simulink model "CCMVgaMotor"  (R2022b)
settings: Solver=FixedStepDiscrete  StartTime=0.0  StopTime=10.0  FixedStep=0.01  RelTol=1e-3
model workspace (MAT data in the model file):
  NvMBlockVgaMtrCalData = Simulink.Signal
  NvMBlockVgaMtrCalData.DataType = 'UInt8'
  NvMBlockVgaMtrCalData.Dimensions = [210 1]
  NvMBlockVgaMtrCalData.DimensionsMode = 'auto'
  NvMBlockVgaMtrCalData.Complexity = 'real'
  NvMBlockVgaMtrCalData.SampleTime = -1
  NvMBlockVgaMtrCalData.SamplingMode = 'auto'
  NvMBlockVgaMtrCalData.InitialValue = 'NvMBlockVgaMtrCalDataInit'
  NvMBlockVgaMtrCalDataInit = Simulink.Parameter
  NvMBlockVgaMtrCalDataInit.Value = [0; 0; 0; 0; 0; 0; 0; 0; 0; 0; 0; 0; 0; 0; 0; 0; 0; 0; 0; 0; 0; 0; 0; 0; 0; 0; 0; 0; 0; 0; 0; 0; 0; 0; 0; 0; 0; 0; 0; 0; 0; 0; 0; 0; 0; 0; 0; 0; 0; 0; 0; 0; 0; 0; 0; 0; 0; 0; 0; 0; 0; 0; 0; 0; 0; 0; 0; 0; 0; 0; 0; 0; 0; 0; 0; 0; 0; 0; 0; 0; 0; 0; 0; 0; 0; 0; 0; 0; 0; 0; 0; 0; 0; 0; 0; 0; 0; 0; 0; 0; 0; 0; 0; 0; 0; 0; 0; 0; 0; 0; 0; 0; 0; 0; 0; 0; 0; 0; 0; 0; 0; 0; 0; 0; 0; 0; 0; 0; 0; 0; 0; 0; 0; 0; 0; 0; 0; 0; 0; 0; 0; 0; 0; 0; 0; 0; 0; 0; 0; 0; 0; 0; 0; 0; 0; 0; 0; 0; 0; 0; 0; 0; 0; 0; 0; 0; 0; 0; 0; 0; 0; 0; 0; 0; 0; 0; 0; 0; 0; 0; 0; 0; 0; 0; 0; 0; 0; 0; 0; 0; 0; 0; 0; 0; 0; 0; 0; 0; 0; 0; 0; 0; 0; 0; 0; 0; 0; 0; 0; 0]
  NvMBlockVgaMtrCalDataInit.DataType = 'UInt8'
  NvMBlockVgaMtrCalDataInit.Complexity = 'real'
  NvMBlockVgaMtrCalDataInit.Dimensions = [210 1]
  VgaWakeUpFlag = Simulink.Signal
  VgaWakeUpFlag.DataType = 'UInt8'
  VgaWakeUpFlag.Dimensions = 1
  VgaWakeUpFlag.DimensionsMode = 'auto'
  VgaWakeUpFlag.Complexity = 'real'
  VgaWakeUpFlag.SampleTime = -1
  VgaWakeUpFlag.SamplingMode = 'auto'
undefined symbols (not defined in the file or in its folder): ComChlType, DioChannelType, LinFrameType, NetworkChlType, NvMBlockId, P_DioLevelType, P_LinIfSchHandleType, P_Std_ReturnType, PowerChlType, VGAChlType
(part 1 of 4)
settings Model: Creator=blue.ge  LastModifiedBy=blue.ge
settings DataIOCC: SaveFormat=StructureWithTime
settings OptimizationCC: DefaultParameterBehavior=Inlined  EnhancedBackFolding=on  PassReuseOutputArgsAs=Individual arguments  ZeroExternalMemoryAtStartup=off  ZeroInternalMemoryAtStartup=off  OptimizeBlockOrder=speed  DifferentSizesBufferReuse=on  OptimizationCustomize=off  EfficientTunableParamExpr=on
settings DebuggingCC: ParameterTunabilityLossMsg=error
settings ModelReferenceCC: ModelReferenceNumInstancesAllowed=Single
settings SFSimCC: MATLABDynamicMemAlloc=off
settings RTWCC: Description=AUTOSAR  SystemTargetFile=autosar.tlc  GenCodeOnly=on  TemplateMakefile=ert_default_tmf
settings CodeAppCC: ForceParamTrailComments=on  IgnoreCustomStorageClasses=off  OperatorAnnotations=on  SimulinkDataObjDesc=on  SFDataObjDesc=on  InsertBlockDesc=on
settings STFCustomTargetCC: TargetLangStandard=C99 (ISO)  MultiwordLength=256  ParMdlRefBuildCompliant=on  CompOptLevelCompliant=on  IncludeMdlTerminateFcn=off  CombineOutputUpdateFcns=on  SuppressErrorStatus=on  ERTCustomFileBanners=on  SupportNonFinite=off  SupportComplex=off  SupportContinuousTime=off  SupportNonInlinedSFcns=off  RemoveResetFunc=on  MATLABClassNameForMDSCustomization=Simulink.SoftwareTarget.ERTCustomization  AutosarCompliant=on  UseToolchainInfoCompliant=on  LookupTableObjectStructAxisOrder=2,1,3,4,...  LUTObjectStructOrderEvenSpacing=Size,Table,Breakpoints  SystemTargetFile=autosar.tlc
settings ERTTargetCC: TargetLangStandard=C99 (ISO)  ConcurrentExecutionCompliant=off  IncludeMdlTerminateFcn=off  SuppressErrorStatus=on  SupportNonFinite=off  SupportComplex=off  ModelStepFunctionPrototypeControlCompliant=off  CPPClassGenCompliant=off  AutosarCompliant=on  LookupTableObjectStructAxisOrder=2,1,3,4,...  LUTObjectStructOrderEvenSpacing=Size,Table,Breakpoints  InlinedParameterPlacement=NonHierarchical  ERTCustomFileTemplate=example_file_process.tlc

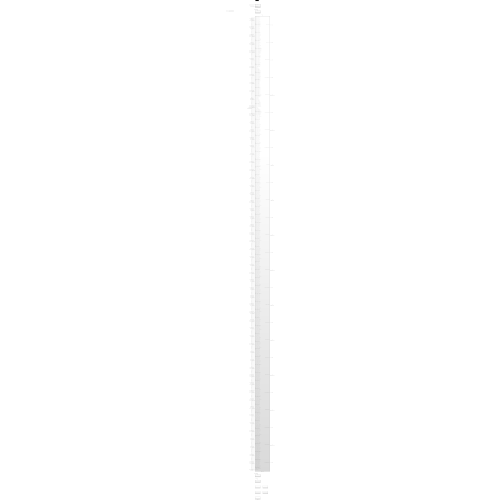
[thumbnail: Simulink's stored preview of the top-level diagram]
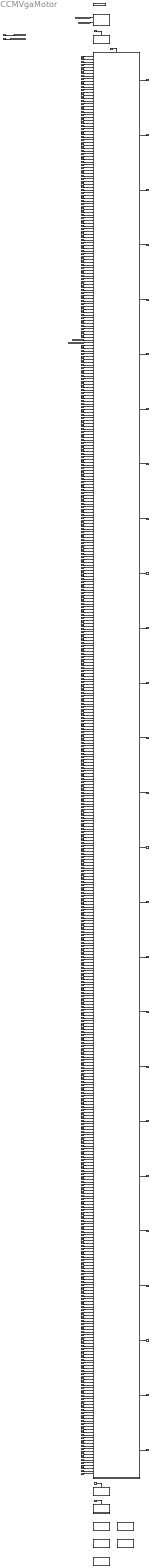
== system CCMVgaMotor ==
  CCMVgaMotor_50msRunnable : Inport  SampleTime=0.05  OutputFunctionCall=on
  CCMVgaMotor_Runnable : Inport  Port=2  SampleTime=0.02  OutputFunctionCall=on
  CCMVGAMotor_VentnMotAdr : Inport  Port=3  OutputFunctionCall=on
  CCMVGAMotor_VentnMotAdr2 : Inport  Port=4  OutputFunctionCall=on
  SbcWakeupSta_SbcWakeupSta : Inport  Port=5  OutDataTypeStr=Enum: SbcWakeupSta  PortDimensions=1
  SbcPwrOn_SbcPwrOn : Inport  Port=6  OutDataTypeStr=PwrOnff  PortDimensions=1
  CarCfgForClimaCtrl_CarCfgForAirClng : Inport  Port=7  OutDataTypeStr=Enum: CarCfgForAirClng  PortDimensions=1
  CarCfgForClimaCtrl_CarCfgForAirClnrAdv : Inport  Port=8  OutDataTypeStr=UInt8  PortDimensions=1
  CarCfgForClimaCtrl_CarCfgForAirFrag : Inport  Port=9  OutDataTypeStr=Enum: CarCfgForAirFrag  PortDimensions=1
  CarCfgForClimaCtrl_CarCfgForAirMtrlSnsr : Inport  Port=10  OutDataTypeStr=Enum: CarCfgForAirMtrlSnsrGly  PortDimensions=1
  CarCfgForClimaCtrl_CarCfgForAirQly : Inport  Port=11  OutDataTypeStr=Enum: CarCfgForAirQlyGly  PortDimensions=1
  CarCfgForClimaCtrl_CarCfgForAmntOfSeat : Inport  Port=12  OutDataTypeStr=Enum: CarCfgForAmntOfSeatTyp3  PortDimensions=1
  CarCfgForClimaCtrl_CarCfgForAuxWtrPmp : Inport  Port=13  OutDataTypeStr=Enum: CarCfgForAuxWtrPmp  PortDimensions=1
  CarCfgForClimaCtrl_CarCfgForBypVlvInHvac : Inport  Port=14  OutDataTypeStr=Enum: CarCfgForBypVlvInHvac2  PortDimensions=1
  CarCfgForClimaCtrl_CarCfgForClsVlvInHvac : Inport  Port=15  OutDataTypeStr=Enum: CarCfgForClsVlvInHvac  PortDimensions=1
  CarCfgForClimaCtrl_CarCfgForCmprAcTyp : Inport  Port=16  OutDataTypeStr=UInt8  PortDimensions=1
  CarCfgForClimaCtrl_CarCfgForCmptmtClng : Inport  Port=17  OutDataTypeStr=Enum: CarCfgForCmptmtClng  PortDimensions=1
  CarCfgForClimaCtrl_CarCfgForElecVentAdjmt : Inport  Port=18  OutDataTypeStr=Enum: CarCfgForElecVentAdjmt  PortDimensions=1
  CarCfgForClimaCtrl_CarCfgForFlcTyp : Inport  Port=19  OutDataTypeStr=Enum: CarCfgForFlcTyp  PortDimensions=1
  CarCfgForClimaCtrl_CarCfgForFoldCSD : Inport  Port=20  OutDataTypeStr=Enum: CarCfgForFoldCSD  PortDimensions=1
  CarCfgForClimaCtrl_CarCfgForFuTyp : Inport  Port=21  OutDataTypeStr=Enum: CarCfgForFuTyp  PortDimensions=1
  CarCfgForClimaCtrl_CarCfgForGlassTyp : Inport  Port=22  OutDataTypeStr=Enum: CarCfgForGlassTyp  PortDimensions=1
  CarCfgForClimaCtrl_CarCfgForHeatPmp : Inport  Port=23  OutDataTypeStr=Enum: CarCfgForHeatPmpTyp  PortDimensions=1
  CarCfgForClimaCtrl_CarCfgForHeatrDurgDrvg : Inport  Port=24  OutDataTypeStr=Enum: CarCfgForHeatrDurgDrvg  PortDimensions=1
  CarCfgForClimaCtrl_CarCfgForHeatrTyp : Inport  Port=25  OutDataTypeStr=UInt8  PortDimensions=1
  CarCfgForClimaCtrl_CarCfgForHvacFilTyp : Inport  Port=26  OutDataTypeStr=Enum: CarCfgForHvacFilTypGly  PortDimensions=1
  CarCfgForClimaCtrl_CarCfgForHvacFlrSnsrForT : Inport  Port=27  OutDataTypeStr=Enum: CarCfgForHvacFlrSnsrForT  PortDimensions=1
  CarCfgForClimaCtrl_CarCfgForHvacHeatrSnsrT : Inport  Port=28  OutDataTypeStr=Enum: CarCfgForHvacHeatrSnsrT  PortDimensions=1
  CarCfgForClimaCtrl_CarCfgForHvacRe : Inport  Port=29  OutDataTypeStr=UInt8  PortDimensions=1
  CarCfgForClimaCtrl_CarCfgForHvacTyp : Inport  Port=30  OutDataTypeStr=UInt8  PortDimensions=1
  CarCfgForClimaCtrl_CarCfgForLoVoltElecCfg : Inport  Port=31  OutDataTypeStr=Enum: CarCfgForLoVoltElecCfg  PortDimensions=1
  CarCfgForClimaCtrl_CarCfgForNavSysandIntnmap : Inport  Port=32  OutDataTypeStr=Enum: CarCfgForNavSysandIntnmap  PortDimensions=1
  CarCfgForClimaCtrl_CarCfgForNrOfDoor : Inport  Port=33  OutDataTypeStr=Enum: CarCfgForNrOfDoor2  PortDimensions=1
  CarCfgForClimaCtrl_CarCfgForPipeInFuTank : Inport  Port=34  OutDataTypeStr=Enum: CarCfgForPipeInFuTank  PortDimensions=1
  CarCfgForClimaCtrl_CarCfgForPreClng : Inport  Port=35  OutDataTypeStr=Enum: CarCfgForPreClng  PortDimensions=1
  CarCfgForClimaCtrl_CarCfgForPrkgCDSNotAvl : Inport  Port=36  OutDataTypeStr=Enum: CarCfgForPrkgCDSNotAvl  PortDimensions=1
  CarCfgForClimaCtrl_CarCfgForPrkgClima : Inport  Port=37  OutDataTypeStr=Enum: CarCfgForPrkgClima  PortDimensions=1
  CarCfgForClimaCtrl_CarCfgForPrpsnTyp : Inport  Port=38  OutDataTypeStr=Enum: CarCfgForPrpsnTypGly  PortDimensions=1
  CarCfgForClimaCtrl_CarCfgForRemEngStrtPreCdn : Inport  Port=39  OutDataTypeStr=Enum: CarCfgForRemEngStrtPreCdn  PortDimensions=1
  CarCfgForClimaCtrl_CarCfgForRoofHatch : Inport  Port=40  OutDataTypeStr=Enum: CarCfgForRoofHatch  PortDimensions=1
  CarCfgForClimaCtrl_CarCfgForSeatHeatgCal : Inport  Port=41  OutDataTypeStr=Enum: CarCfgForSeatHeatgCal1  PortDimensions=1
  CarCfgForClimaCtrl_CarCfgForSeatHeatgForRowFirst : Inport  Port=42  OutDataTypeStr=Enum: CarCfgForSeatHeatgForRowFirst  PortDimensions=1
  CarCfgForClimaCtrl_CarCfgForSeatHeatgForRowSec : Inport  Port=43  OutDataTypeStr=Enum: CarCfgForSeatHeatgForRowSec  PortDimensions=1
  CarCfgForClimaCtrl_CarCfgForSeatVentnForRowFirst : Inport  Port=44  OutDataTypeStr=Enum: CarCfgForSeatVentnForRowFirst1  PortDimensions=1
  CarCfgForClimaCtrl_CarCfgForSeatVentnForRowSec : Inport  Port=45  OutDataTypeStr=Enum: CarCfgForSeatVentnForRowSecGly  PortDimensions=1
  CarCfgForClimaCtrl_CarCfgForSecRowVentnCenter : Inport  Port=46  OutDataTypeStr=Enum: CarCfgForSecRowVentnCenter  PortDimensions=1
  CarCfgForClimaCtrl_CarCfgForSecRowVentnSide : Inport  Port=47  OutDataTypeStr=Enum: CarCfgForSecRowVentnSide  PortDimensions=1
  CarCfgForClimaCtrl_CarCfgForSmallFlowCyc : Inport  Port=48  OutDataTypeStr=Enum: CarCfgForSmallFlowCyc  PortDimensions=1
  CarCfgForClimaCtrl_CarCfgForSmkSnsr : Inport  Port=49  OutDataTypeStr=Enum: CarCfgForSmkSnsr  PortDimensions=1
  CarCfgForClimaCtrl_CarCfgForSnsrForCmptmtT : Inport  Port=50  OutDataTypeStr=Enum: CarCfgForSnsrForCmptmtT  PortDimensions=1
  CarCfgForClimaCtrl_CarCfgForSnsrHumPrsnt : Inport  Port=51  OutDataTypeStr=Enum: CarCfgForSnsrHumPrsnt  PortDimensions=1
  CarCfgForClimaCtrl_CarCfgForSteerWhlHeatg : Inport  Port=52  OutDataTypeStr=UInt8  PortDimensions=1
  CarCfgForClimaCtrl_CarCfgForSteerWhlPosn : Inport  Port=53  OutDataTypeStr=Enum: CarCfgForSteerWhlPosn  PortDimensions=1
  CarCfgForClimaCtrl_CarCfgForSunSnsr : Inport  Port=54  OutDataTypeStr=Enum: CarCfgForSunSnsr  PortDimensions=1
  CarCfgForClimaCtrl_CarCfgForTelmNodTyp : Inport  Port=55  OutDataTypeStr=Enum: CarCfgForTelmNodTyp  PortDimensions=1
  CarCfgForClimaCtrl_CarCfgForThermMngt : Inport  Port=56  OutDataTypeStr=Enum: CarCfgForThermMngt  PortDimensions=1
  CarCfgForClimaCtrl_CarCfgForVehTyp : Inport  Port=57  OutDataTypeStr=Enum: CarCfgForVehTyp3  PortDimensions=1
  CarCfgForClimaCtrl_CarCfgForVgaMotorExistSts : Inport  Port=58  OutDataTypeStr=CarCfgForVgaMotorExistSts  PortDimensions=1
  CarCfgForClimaCtrl_CarCfgForWindHeatd : Inport  Port=59  OutDataTypeStr=Enum: CarCfgForWindHeatd  PortDimensions=1
  CarCfgForClimaCtrl_CarCfgForAutDemst : Inport  Port=60  OutDataTypeStr=UInt8  PortDimensions=1
  CarCfgForClimaCtrl_CarCfgForExtVentnClimaCtrl : Inport  Port=61  OutDataTypeStr=Enum: CarCfgForExtVentnClimaCtrl  PortDimensions=1
  CarCfgForClimaCtrl_CarCfgForStartDevice : Inport  Port=62  OutDataTypeStr=Enum: StartDevice  PortDimensions=1
  HmiElecAirSwngReq_HmiElecAirSwngReq : Inport  Port=63  OutDataTypeStr=Bus: HmiElecAirSwngReq  PortDimensions=1  BusOutputAsStruct=on
  LIN2ScheTableReq_Val : Inport  Port=64  OutDataTypeStr=UInt8  PortDimensions=1
  SysServCarCfgSts_SysServCarID : Inport  Port=65  OutDataTypeStr=Enum: SysServCarID  PortDimensions=1
  SysServCarCfgSts_SysServCCPAQSSts : Inport  Port=66  OutDataTypeStr=UInt8  PortDimensions=1
  SysServCarCfgSts_SysServCCPHEXSensorSts : Inport  Port=67  OutDataTypeStr=UInt8  PortDimensions=1
  SysServCarCfgSts_SysServCCPLin2AFUSts : Inport  Port=68  OutDataTypeStr=Boolean  PortDimensions=1
  SysServCarCfgSts_SysServCCPLin2AIUSts : Inport  Port=69  OutDataTypeStr=UInt8  PortDimensions=1
  SysServCarCfgSts_SysServCCPLin2CDSSts : Inport  Port=70  OutDataTypeStr=Boolean  PortDimensions=1
  SysServCarCfgSts_SysServCCPLin2PMSISts : Inport  Port=71  OutDataTypeStr=Boolean  PortDimensions=1
  SysServCarCfgSts_SysServCCPLin3VGASts : Inport  Port=72  OutDataTypeStr=Boolean  PortDimensions=1
  SysServCarCfgSts_SysServDUAirVentSnrSts : Inport  Port=73  OutDataTypeStr=Boolean  PortDimensions=1
  SysServCarCfgSts_SysServDUBlowerPwmSignSts : Inport  Port=74  OutDataTypeStr=Boolean  PortDimensions=1
  SysServCarCfgSts_SysServDUBlowerPwmSts : Inport  Port=75  OutDataTypeStr=Boolean  PortDimensions=1
  SysServCarCfgSts_SysServDUDCDefrostSts : Inport  Port=76  OutDataTypeStr=UInt8  PortDimensions=1
  SysServCarCfgSts_SysServDUDCModeSts : Inport  Port=77  OutDataTypeStr=UInt8  PortDimensions=1
  SysServCarCfgSts_SysServDUDCRecSts : Inport  Port=78  OutDataTypeStr=UInt8  PortDimensions=1
  SysServCarCfgSts_SysServDUDCSModeSts : Inport  Port=79  OutDataTypeStr=UInt8  PortDimensions=1
  SysServCarCfgSts_SysServDUDCSTLSts : Inport  Port=80  OutDataTypeStr=UInt8  PortDimensions=1
  SysServCarCfgSts_SysServDUDCTLSts : Inport  Port=81  OutDataTypeStr=UInt8  PortDimensions=1
  SysServCarCfgSts_SysServDUDCTRSts : Inport  Port=82  OutDataTypeStr=UInt8  PortDimensions=1
  SysServCarCfgSts_SysServDUFLFootSts : Inport  Port=83  OutDataTypeStr=UInt8  PortDimensions=1
  SysServCarCfgSts_SysServDUFLVentSts : Inport  Port=84  OutDataTypeStr=UInt8  PortDimensions=1
  SysServCarCfgSts_SysServDUFRFootSts : Inport  Port=85  OutDataTypeStr=UInt8  PortDimensions=1
  SysServCarCfgSts_SysServDUFRVentSts : Inport  Port=86  OutDataTypeStr=UInt8  PortDimensions=1
  SysServCarCfgSts_SysServDUIncarMotorSts : Inport  Port=87  OutDataTypeStr=Boolean  PortDimensions=1
  SysServCarCfgSts_SysServDULin1HBMFSts : Inport  Port=88  OutDataTypeStr=Boolean  PortDimensions=1
  SysServCarCfgSts_SysServDULin4HFAFSts : Inport  Port=89  OutDataTypeStr=UInt32  PortDimensions=1
  SysServCarCfgSts_SysServDUSFLFootSts : Inport  Port=90  OutDataTypeStr=UInt8  PortDimensions=1
  SysServCarCfgSts_SysServDUSFLVentSts : Inport  Port=91  OutDataTypeStr=UInt8  PortDimensions=1
  SysServCarCfgSts_SysServDUSFRFootSts : Inport  Port=92  OutDataTypeStr=UInt8  PortDimensions=1
  SysServCarCfgSts_SysServDUSFRVentSts : Inport  Port=93  OutDataTypeStr=UInt8  PortDimensions=1
  SysServCarCfgSts_SysServDUSnsrForFCmptmtTSts : Inport  Port=94  OutDataTypeStr=UInt8  PortDimensions=1
  SysServCarCfgSts_SysServDUSnsrForRair : Inport  Port=95  OutDataTypeStr=UInt8  PortDimensions=1
  SysServCarCfgSts_SysServDUSnsrForRCmptmtTSts : Inport  Port=96  OutDataTypeStr=UInt8  PortDimensions=1
  VehModMngtGlbSafe1_VehModMngtGlbSafe1 : Inport  Port=97  OutDataTypeStr=Bus: VehModMngtGlbSafe1  PortDimensions=1  BusOutputAsStruct=on
  BatLoadBtrMSts_BatLoadBtrMSts : Inport  Port=98  OutDataTypeStr=Enum: BatLoadBtrMStsEnum  PortDimensions=1
  BatSysBtrMSts_BatSysBtrMSts : Inport  Port=99  OutDataTypeStr=Enum: BatBtrMStsEnum  PortDimensions=1
  VgaMotSetPowerOnInit_PowerOnInit : Inport  Port=100  OutDataTypeStr=Boolean  PortDimensions=1
  CarCfgForClimaCtrl_CarCfgForBRAND : Inport  Port=101  OutDataTypeStr=UInt8  PortDimensions=1
  CarCfgForClimaCtrl_CarCfgForHeadRadar : Inport  Port=102  OutDataTypeStr=Enum: HeadRadar  PortDimensions=1
  CarCfgForClimaCtrl_CarCfgForInteriorViewCamera : Inport  Port=103  OutDataTypeStr=Enum: CarCfgForInteriorViewCamera  PortDimensions=1
  CarCfgForClimaCtrl_CarCfgForLifeDetection : Inport  Port=104  OutDataTypeStr=Enum: LifeDetection  PortDimensions=1
  CarCfgForClimaCtrl_CarCfgForPowerSeats : Inport  Port=105  OutDataTypeStr=Enum: CarCfgForPowerSeats  PortDimensions=1
  CarCfgForClimaCtrl_CarCfgForPTCInFrontHVAC : Inport  Port=106  OutDataTypeStr=UInt8  PortDimensions=1
  CarCfgForClimaCtrl_CarCfgForVentLiftMotorExiSts : Inport  Port=107  OutDataTypeStr=UInt32  PortDimensions=1
  CarCfgForClimaCtrl_CarCfgForWadingAssistSystem : Inport  Port=108  OutDataTypeStr=UInt8  PortDimensions=1
  SysServCarCfgSts_SysServCCPBGMNum : Inport  Port=109  OutDataTypeStr=UInt8  PortDimensions=1
  SysServCarCfgSts_SysServCCPLin2AQSSts : Inport  Port=110  OutDataTypeStr=Boolean  PortDimensions=1
  SysServCarCfgSts_SysServDUBlowerPwmSignTypeSts : Inport  Port=111  OutDataTypeStr=UInt8  PortDimensions=1
  SysServCarCfgSts_SysServDUDCFLMdeSts : Inport  Port=112  OutDataTypeStr=UInt8  PortDimensions=1
  SysServCarCfgSts_SysServDUReBlowerPwmSignSts : Inport  Port=113  OutDataTypeStr=UInt8  PortDimensions=1
  SysServCarCfgSts_SysServDUReBlowerPwmSts : Inport  Port=114  OutDataTypeStr=UInt8  PortDimensions=1
  SysServCarCfgSts_SysServLowSideRETXVSts : Inport  Port=115  OutDataTypeStr=UInt8  PortDimensions=1
  VentnActr01ActPson_VentnActr01ActPson : Inport  Port=116  OutDataTypeStr=UInt16  PortDimensions=1
  VentnActr01ActPson_VentnActr01ActPson_IsUpdated : Inport  Port=117  OutDataTypeStr=boolean  PortDimensions=1
  VentnActr01BlckInd_VentnActr01BlckInd : Inport  Port=118  OutDataTypeStr=Enum: VentnActr01BlckInd  PortDimensions=1
  VentnActr01BlckSts_VentnActr01BlckSts : Inport  Port=119  OutDataTypeStr=Enum: VentnActr01BlckSts  PortDimensions=1
  VentnActr01BusAdr_VentnActr01BusAdr : Inport  Port=120  OutDataTypeStr=UInt8  PortDimensions=1
  VentnActr01CoilSts_VentnActr01CoilSts : Inport  Port=121  OutDataTypeStr=Enum: VentnMotUrgcUnlck  PortDimensions=1
  VentnActr01DrvDir_VentnActr01DrvDir : Inport  Port=122  OutDataTypeStr=Enum: VentnActr01DrvDir  PortDimensions=1
  VentnActr01ElecErr_VentnActr01ElecErr : Inport  Port=123  OutDataTypeStr=Enum: VentnActr01ElecErr  PortDimensions=1
  VentnActr01Mode_VentnActr01Mode : Inport  Port=124  OutDataTypeStr=Enum: VentnMotMode  PortDimensions=1
  VentnActr01MovDir_VentnActr01MovDir : Inport  Port=125  OutDataTypeStr=Enum: VentnMotDir  PortDimensions=1
  VentnActr01OvrTempErr_VentnActr01OvrTempErr : Inport  Port=126  OutDataTypeStr=Enum: VentnActr01OvrTempErr  PortDimensions=1
  VentnActr01PsonSts_VentnActr01PsonSts : Inport  Port=127  OutDataTypeStr=Enum: VentnMotPsonAvl  PortDimensions=1
  VentnActr01ResetSts_VentnActr01ResetSts : Inport  Port=128  OutDataTypeStr=Enum: VentnActr01ResetSts  PortDimensions=1
  VentnActr01SpclFct_VentnActr01SpclFct : Inport  Port=129  OutDataTypeStr=Enum: VentnActr01SpclFct  PortDimensions=1
  VentnActr01SpdSts_VentnActr01SpdSts : Inport  Port=130  OutDataTypeStr=Enum: VentnActr01SpdSts  PortDimensions=1
  VentnActr01TrqSts_VentnActr01TrqSts : Inport  Port=131  OutDataTypeStr=Enum: VentnMotUrgcUnlck  PortDimensions=1
  VentnActr01UrgcExist_VentnActr01UrgcExist : Inport  Port=132  OutDataTypeStr=Enum: VentnActr01UrgcExist  PortDimensions=1
  VentnActr01UrgcPson_VentnActr01UrgcPson : Inport  Port=133  OutDataTypeStr=Enum: VentnMotUrgcPson  PortDimensions=1
  VentnActr01UrgcSts_VentnActr01UrgcSts : Inport  Port=134  OutDataTypeStr=Enum: VentnMotUrgcUnlck  PortDimensions=1
  VentnActr01VoltErr_VentnActr01VoltErr : Inport  Port=135  OutDataTypeStr=Enum: VentnActr01VoltErr  PortDimensions=1
  VentnActr02ActPson_VentnActr02ActPson : Inport  Port=136  OutDataTypeStr=UInt16  PortDimensions=1
  VentnActr02ActPson_VentnActr02ActPson_IsUpdated : Inport  Port=137  OutDataTypeStr=boolean  PortDimensions=1
  VentnActr02BlckInd_VentnActr02BlckInd : Inport  Port=138  OutDataTypeStr=Enum: VentnActr01BlckInd  PortDimensions=1
  VentnActr02BlckSts_VentnActr02BlckSts : Inport  Port=139  OutDataTypeStr=Enum: VentnActr01BlckSts  PortDimensions=1
  VentnActr02BusAdr_VentnActr02BusAdr : Inport  Port=140  OutDataTypeStr=UInt8  PortDimensions=1
  VentnActr02CoilSts_VentnActr02CoilSts : Inport  Port=141  OutDataTypeStr=Enum: VentnMotUrgcUnlck  PortDimensions=1
  VentnActr02DrvDir_VentnActr02DrvDir : Inport  Port=142  OutDataTypeStr=Enum: VentnActr01DrvDir  PortDimensions=1
  VentnActr02ElecErr_VentnActr02ElecErr : Inport  Port=143  OutDataTypeStr=Enum: VentnActr01ElecErr  PortDimensions=1
  VentnActr02Mode_VentnActr02Mode : Inport  Port=144  OutDataTypeStr=Enum: VentnMotMode  PortDimensions=1
  VentnActr02MovDir_VentnActr02MovDir : Inport  Port=145  OutDataTypeStr=Enum: VentnMotDir  PortDimensions=1
  VentnActr02OvrTempErr_VentnActr02OvrTempErr : Inport  Port=146  OutDataTypeStr=Enum: VentnActr01OvrTempErr  PortDimensions=1
  VentnActr02PsonSts_VentnActr02PsonSts : Inport  Port=147  OutDataTypeStr=Enum: VentnMotPsonAvl  PortDimensions=1
  VentnActr02ResetSts_VentnActr02ResetSts : Inport  Port=148  OutDataTypeStr=Enum: VentnActr01ResetSts  PortDimensions=1
  VentnActr02SpclFct_VentnActr02SpclFct : Inport  Port=149  OutDataTypeStr=Enum: VentnActr01SpclFct  PortDimensions=1
  VentnActr02SpdSts_VentnActr02SpdSts : Inport  Port=150  OutDataTypeStr=Enum: VentnActr01SpdSts  PortDimensions=1
  VentnActr02TrqSts_VentnActr02TrqSts : Inport  Port=151  OutDataTypeStr=Enum: VentnMotUrgcUnlck  PortDimensions=1
  VentnActr02UrgcExist_VentnActr02UrgcExist : Inport  Port=152  OutDataTypeStr=Enum: VentnActr01UrgcExist  PortDimensions=1
  VentnActr02UrgcPson_VentnActr02UrgcPson : Inport  Port=153  OutDataTypeStr=Enum: VentnMotUrgcPson  PortDimensions=1
  VentnActr02UrgcSts_VentnActr02UrgcSts : Inport  Port=154  OutDataTypeStr=Enum: VentnMotUrgcUnlck  PortDimensions=1
  VentnActr02VoltErr_VentnActr02VoltErr : Inport  Port=155  OutDataTypeStr=Enum: VentnActr01VoltErr  PortDimensions=1
  VentnActr03ActPson_VentnActr03ActPson : Inport  Port=156  OutDataTypeStr=UInt16  PortDimensions=1
  VentnActr03ActPson_VentnActr03ActPson_IsUpdated : Inport  Port=157  OutDataTypeStr=boolean  PortDimensions=1
  VentnActr03BlckInd_VentnActr03BlckInd : Inport  Port=158  OutDataTypeStr=Enum: VentnActr01BlckInd  PortDimensions=1
  VentnActr03BlckSts_VentnActr03BlckSts : Inport  Port=159  OutDataTypeStr=Enum: VentnActr01BlckSts  PortDimensions=1
  VentnActr03BusAdr_VentnActr03BusAdr : Inport  Port=160  OutDataTypeStr=UInt8  PortDimensions=1
  VentnActr03CoilSts_VentnActr03CoilSts : Inport  Port=161  OutDataTypeStr=Enum: VentnMotUrgcUnlck  PortDimensions=1
  VentnActr03DrvDir_VentnActr03DrvDir : Inport  Port=162  OutDataTypeStr=Enum: VentnActr01DrvDir  PortDimensions=1
  VentnActr03ElecErr_VentnActr03ElecErr : Inport  Port=163  OutDataTypeStr=Enum: VentnActr01ElecErr  PortDimensions=1
  VentnActr03Mode_VentnActr03Mode : Inport  Port=164  OutDataTypeStr=Enum: VentnMotMode  PortDimensions=1
  VentnActr03MovDir_VentnActr03MovDir : Inport  Port=165  OutDataTypeStr=Enum: VentnMotDir  PortDimensions=1
  VentnActr03OvrTempErr_VentnActr03OvrTempErr : Inport  Port=166  OutDataTypeStr=Enum: VentnActr01OvrTempErr  PortDimensions=1
  VentnActr03PsonSts_VentnActr03PsonSts : Inport  Port=167  OutDataTypeStr=Enum: VentnMotPsonAvl  PortDimensions=1
  VentnActr03ResetSts_VentnActr03ResetSts : Inport  Port=168  OutDataTypeStr=Enum: VentnActr01ResetSts  PortDimensions=1
  VentnActr03SpclFct_VentnActr03SpclFct : Inport  Port=169  OutDataTypeStr=Enum: VentnActr01SpclFct  PortDimensions=1
  VentnActr03SpdSts_VentnActr03SpdSts : Inport  Port=170  OutDataTypeStr=Enum: VentnActr01SpdSts  PortDimensions=1
  VentnActr03TrqSts_VentnActr03TrqSts : Inport  Port=171  OutDataTypeStr=Enum: VentnMotUrgcUnlck  PortDimensions=1
  VentnActr03UrgcExist_VentnActr03UrgcExist : Inport  Port=172  OutDataTypeStr=Enum: VentnActr01UrgcExist  PortDimensions=1
  VentnActr03UrgcPson_VentnActr03UrgcPson : Inport  Port=173  OutDataTypeStr=Enum: VentnMotUrgcPson  PortDimensions=1
  VentnActr03UrgcSts_VentnActr03UrgcSts : Inport  Port=174  OutDataTypeStr=Enum: VentnMotUrgcUnlck  PortDimensions=1
  VentnActr03VoltErr_VentnActr03VoltErr : Inport  Port=175  OutDataTypeStr=Enum: VentnActr01VoltErr  PortDimensions=1
  VentnActr04ActPson_VentnActr04ActPson : Inport  Port=176  OutDataTypeStr=UInt16  PortDimensions=1
  VentnActr04ActPson_VentnActr04ActPson_IsUpdated : Inport  Port=177  OutDataTypeStr=boolean  PortDimensions=1
  VentnActr04BlckInd_VentnActr04BlckInd : Inport  Port=178  OutDataTypeStr=Enum: VentnActr01BlckInd  PortDimensions=1
  VentnActr04BlckSts_VentnActr04BlckSts : Inport  Port=179  OutDataTypeStr=Enum: VentnActr01BlckSts  PortDimensions=1
  VentnActr04BusAdr_VentnActr04BusAdr : Inport  Port=180  OutDataTypeStr=UInt8  PortDimensions=1
  VentnActr04CoilSts_VentnActr04CoilSts : Inport  Port=181  OutDataTypeStr=Enum: VentnMotUrgcUnlck  PortDimensions=1
  VentnActr04DrvDir_VentnActr04DrvDir : Inport  Port=182  OutDataTypeStr=Enum: VentnActr01DrvDir  PortDimensions=1
  VentnActr04ElecErr_VentnActr04ElecErr : Inport  Port=183  OutDataTypeStr=Enum: VentnActr01ElecErr  PortDimensions=1
  VentnActr04Mode_VentnActr04Mode : Inport  Port=184  OutDataTypeStr=Enum: VentnMotMode  PortDimensions=1
  VentnActr04MovDir_VentnActr04MovDir : Inport  Port=185  OutDataTypeStr=Enum: VentnMotDir  PortDimensions=1
  VentnActr04OvrTempErr_VentnActr04OvrTempErr : Inport  Port=186  OutDataTypeStr=Enum: VentnActr01OvrTempErr  PortDimensions=1
  VentnActr04PsonSts_VentnActr04PsonSts : Inport  Port=187  OutDataTypeStr=Enum: VentnMotPsonAvl  PortDimensions=1
  VentnActr04ResetSts_VentnActr04ResetSts : Inport  Port=188  OutDataTypeStr=Enum: VentnActr01ResetSts  PortDimensions=1
  VentnActr04SpclFct_VentnActr04SpclFct : Inport  Port=189  OutDataTypeStr=Enum: VentnActr01SpclFct  PortDimensions=1
  VentnActr04SpdSts_VentnActr04SpdSts : Inport  Port=190  OutDataTypeStr=Enum: VentnActr01SpdSts  PortDimensions=1
  VentnActr04TrqSts_VentnActr04TrqSts : Inport  Port=191  OutDataTypeStr=Enum: VentnMotUrgcUnlck  PortDimensions=1
  VentnActr04UrgcExist_VentnActr04UrgcExist : Inport  Port=192  OutDataTypeStr=Enum: VentnActr01UrgcExist  PortDimensions=1
  VentnActr04UrgcPson_VentnActr04UrgcPson : Inport  Port=193  OutDataTypeStr=Enum: VentnMotUrgcPson  PortDimensions=1
  VentnActr04UrgcSts_VentnActr04UrgcSts : Inport  Port=194  OutDataTypeStr=Enum: VentnMotUrgcUnlck  PortDimensions=1
  VentnActr04VoltErr_VentnActr04VoltErr : Inport  Port=195  OutDataTypeStr=Enum: VentnActr01VoltErr  PortDimensions=1
  VentnActr05ActPson_VentnActr05ActPson : Inport  Port=196  OutDataTypeStr=UInt16  PortDimensions=1
  VentnActr05ActPson_VentnActr05ActPson_IsUpdated : Inport  Port=197  OutDataTypeStr=boolean  PortDimensions=1
  VentnActr05BlckInd_VentnActr05BlckInd : Inport  Port=198  OutDataTypeStr=Enum: VentnActr01BlckInd  PortDimensions=1
  VentnActr05BlckSts_VentnActr05BlckSts : Inport  Port=199  OutDataTypeStr=Enum: VentnActr01BlckSts  PortDimensions=1
  VentnActr05BusAdr_VentnActr05BusAdr : Inport  Port=200  OutDataTypeStr=UInt8  PortDimensions=1
  VentnActr05CoilSts_VentnActr05CoilSts : Inport  Port=201  OutDataTypeStr=Enum: VentnMotUrgcUnlck  PortDimensions=1
  VentnActr05DrvDir_VentnActr05DrvDir : Inport  Port=202  OutDataTypeStr=Enum: VentnActr01DrvDir  PortDimensions=1
  VentnActr05ElecErr_VentnActr05ElecErr : Inport  Port=203  OutDataTypeStr=Enum: VentnActr01ElecErr  PortDimensions=1
  VentnActr05Mode_VentnActr05Mode : Inport  Port=204  OutDataTypeStr=Enum: VentnMotMode  PortDimensions=1
  VentnActr05MovDir_VentnActr05MovDir : Inport  Port=205  OutDataTypeStr=Enum: VentnMotDir  PortDimensions=1
  VentnActr05OvrTempErr_VentnActr05OvrTempErr : Inport  Port=206  OutDataTypeStr=Enum: VentnActr01OvrTempErr  PortDimensions=1
  VentnActr05PsonSts_VentnActr05PsonSts : Inport  Port=207  OutDataTypeStr=Enum: VentnMotPsonAvl  PortDimensions=1
  VentnActr05ResetSts_VentnActr05ResetSts : Inport  Port=208  OutDataTypeStr=Enum: VentnActr01ResetSts  PortDimensions=1
  VentnActr05SpclFct_VentnActr05SpclFct : Inport  Port=209  OutDataTypeStr=Enum: VentnActr01SpclFct  PortDimensions=1
  VentnActr05SpdSts_VentnActr05SpdSts : Inport  Port=210  OutDataTypeStr=Enum: VentnActr01SpdSts  PortDimensions=1
  VentnActr05TrqSts_VentnActr05TrqSts : Inport  Port=211  OutDataTypeStr=Enum: VentnMotUrgcUnlck  PortDimensions=1
  VentnActr05UrgcExist_VentnActr05UrgcExist : Inport  Port=212  OutDataTypeStr=Enum: VentnActr01UrgcExist  PortDimensions=1
  VentnActr05UrgcPson_VentnActr05UrgcPson : Inport  Port=213  OutDataTypeStr=Enum: VentnMotUrgcPson  PortDimensions=1
  VentnActr05UrgcSts_VentnActr05UrgcSts : Inport  Port=214  OutDataTypeStr=Enum: VentnMotUrgcUnlck  PortDimensions=1
  VentnActr05VoltErr_VentnActr05VoltErr : Inport  Port=215  OutDataTypeStr=Enum: VentnActr01VoltErr  PortDimensions=1
  VentnActr06ActPson_VentnActr06ActPson : Inport  Port=216  OutDataTypeStr=UInt16  PortDimensions=1
  VentnActr06ActPson_VentnActr06ActPson_IsUpdated : Inport  Port=217  OutDataTypeStr=boolean  PortDimensions=1
  VentnActr06BlckInd_VentnActr06BlckInd : Inport  Port=218  OutDataTypeStr=Enum: VentnActr01BlckInd  PortDimensions=1
  VentnActr06BlckSts_VentnActr06BlckSts : Inport  Port=219  OutDataTypeStr=Enum: VentnActr01BlckSts  PortDimensions=1
  VentnActr06BusAdr_VentnActr06BusAdr : Inport  Port=220  OutDataTypeStr=UInt8  PortDimensions=1
  VentnActr06CoilSts_VentnActr06CoilSts : Inport  Port=221  OutDataTypeStr=Enum: VentnMotUrgcUnlck  PortDimensions=1
  VentnActr06DrvDir_VentnActr06DrvDir : Inport  Port=222  OutDataTypeStr=Enum: VentnActr01DrvDir  PortDimensions=1
  VentnActr06ElecErr_VentnActr06ElecErr : Inport  Port=223  OutDataTypeStr=Enum: VentnActr01ElecErr  PortDimensions=1
  VentnActr06Mode_VentnActr06Mode : Inport  Port=224  OutDataTypeStr=Enum: VentnMotMode  PortDimensions=1
  VentnActr06MovDir_VentnActr06MovDir : Inport  Port=225  OutDataTypeStr=Enum: VentnMotDir  PortDimensions=1
  VentnActr06OvrTempErr_VentnActr06OvrTempErr : Inport  Port=226  OutDataTypeStr=Enum: VentnActr01OvrTempErr  PortDimensions=1
  VentnActr06PsonSts_VentnActr06PsonSts : Inport  Port=227  OutDataTypeStr=Enum: VentnMotPsonAvl  PortDimensions=1
  VentnActr06ResetSts_VentnActr06ResetSts : Inport  Port=228  OutDataTypeStr=Enum: VentnActr01ResetSts  PortDimensions=1
  VentnActr06SpclFct_VentnActr06SpclFct : Inport  Port=229  OutDataTypeStr=Enum: VentnActr01SpclFct  PortDimensions=1
  VentnActr06SpdSts_VentnActr06SpdSts : Inport  Port=230  OutDataTypeStr=Enum: VentnActr01SpdSts  PortDimensions=1
  VentnActr06TrqSts_VentnActr06TrqSts : Inport  Port=231  OutDataTypeStr=Enum: VentnMotUrgcUnlck  PortDimensions=1
  VentnActr06UrgcExist_VentnActr06UrgcExist : Inport  Port=232  OutDataTypeStr=Enum: VentnActr01UrgcExist  PortDimensions=1
  VentnActr06UrgcPson_VentnActr06UrgcPson : Inport  Port=233  OutDataTypeStr=Enum: VentnMotUrgcPson  PortDimensions=1
  VentnActr06UrgcSts_VentnActr06UrgcSts : Inport  Port=234  OutDataTypeStr=Enum: VentnMotUrgcUnlck  PortDimensions=1
  VentnActr06VoltErr_VentnActr06VoltErr : Inport  Port=235  OutDataTypeStr=Enum: VentnActr01VoltErr  PortDimensions=1
  VentnActr07ActPson_VentnActr07ActPson : Inport  Port=236  OutDataTypeStr=UInt16  PortDimensions=1
  VentnActr07ActPson_VentnActr07ActPson_IsUpdated : Inport  Port=237  OutDataTypeStr=boolean  PortDimensions=1
  VentnActr07BlckInd_VentnActr07BlckInd : Inport  Port=238  OutDataTypeStr=Enum: VentnActr01BlckInd  PortDimensions=1
  VentnActr07BlckSts_VentnActr07BlckSts : Inport  Port=239  OutDataTypeStr=Enum: VentnActr01BlckSts  PortDimensions=1
  VentnActr07BusAdr_VentnActr07BusAdr : Inport  Port=240  OutDataTypeStr=UInt8  PortDimensions=1
  VentnActr07CoilSts_VentnActr07CoilSts : Inport  Port=241  OutDataTypeStr=Enum: VentnMotUrgcUnlck  PortDimensions=1
  VentnActr07DrvDir_VentnActr07DrvDir : Inport  Port=242  OutDataTypeStr=Enum: VentnActr01DrvDir  PortDimensions=1
  VentnActr07ElecErr_VentnActr07ElecErr : Inport  Port=243  OutDataTypeStr=Enum: VentnActr01ElecErr  PortDimensions=1
  VentnActr07Mode_VentnActr07Mode : Inport  Port=244  OutDataTypeStr=Enum: VentnMotMode  PortDimensions=1
  VentnActr07MovDir_VentnActr07MovDir : Inport  Port=245  OutDataTypeStr=Enum: VentnMotDir  PortDimensions=1
  VentnActr07OvrTempErr_VentnActr07OvrTempErr : Inport  Port=246  OutDataTypeStr=Enum: VentnActr01OvrTempErr  PortDimensions=1
  VentnActr07PsonSts_VentnActr07PsonSts : Inport  Port=247  OutDataTypeStr=Enum: VentnMotPsonAvl  PortDimensions=1
  VentnActr07ResetSts_VentnActr07ResetSts : Inport  Port=248  OutDataTypeStr=Enum: VentnActr01ResetSts  PortDimensions=1
  VentnActr07SpclFct_VentnActr07SpclFct : Inport  Port=249  OutDataTypeStr=Enum: VentnActr01SpclFct  PortDimensions=1
  VentnActr07SpdSts_VentnActr07SpdSts : Inport  Port=250  OutDataTypeStr=Enum: VentnActr01SpdSts  PortDimensions=1
  VentnActr07TrqSts_VentnActr07TrqSts : Inport  Port=251  OutDataTypeStr=Enum: VentnMotUrgcUnlck  PortDimensions=1
  VentnActr07UrgcExist_VentnActr07UrgcExist : Inport  Port=252  OutDataTypeStr=Enum: VentnActr01UrgcExist  PortDimensions=1
  VentnActr07UrgcPson_VentnActr07UrgcPson : Inport  Port=253  OutDataTypeStr=Enum: VentnMotUrgcPson  PortDimensions=1
  VentnActr07UrgcSts_VentnActr07UrgcSts : Inport  Port=254  OutDataTypeStr=Enum: VentnMotUrgcUnlck  PortDimensions=1
  VentnActr07VoltErr_VentnActr07VoltErr : Inport  Port=255  OutDataTypeStr=Enum: VentnActr01VoltErr  PortDimensions=1
  VentnActr08ActPson_VentnActr08ActPson : Inport  Port=256  OutDataTypeStr=UInt16  PortDimensions=1
  VentnActr08ActPson_VentnActr08ActPson_IsUpdated : Inport  Port=257  OutDataTypeStr=boolean  PortDimensions=1
  VentnActr08BlckInd_VentnActr08BlckInd : Inport  Port=258  OutDataTypeStr=Enum: VentnActr01BlckInd  PortDimensions=1
  VentnActr08BlckSts_VentnActr08BlckSts : Inport  Port=259  OutDataTypeStr=Enum: VentnActr01BlckSts  PortDimensions=1
  VentnActr08BusAdr_VentnActr08BusAdr : Inport  Port=260  OutDataTypeStr=UInt8  PortDimensions=1
  VentnActr08CoilSts_VentnActr08CoilSts : Inport  Port=261  OutDataTypeStr=Enum: VentnMotUrgcUnlck  PortDimensions=1
  VentnActr08DrvDir_VentnActr08DrvDir : Inport  Port=262  OutDataTypeStr=Enum: VentnActr01DrvDir  PortDimensions=1
  VentnActr08ElecErr_VentnActr08ElecErr : Inport  Port=263  OutDataTypeStr=Enum: VentnActr01ElecErr  PortDimensions=1
  VentnActr08Mode_VentnActr08Mode : Inport  Port=264  OutDataTypeStr=Enum: VentnMotMode  PortDimensions=1
  VentnActr08MovDir_VentnActr08MovDir : Inport  Port=265  OutDataTypeStr=Enum: VentnMotDir  PortDimensions=1
  VentnActr08OvrTempErr_VentnActr08OvrTempErr : Inport  Port=266  OutDataTypeStr=Enum: VentnActr01OvrTempErr  PortDimensions=1
  VentnActr08PsonSts_VentnActr08PsonSts : Inport  Port=267  OutDataTypeStr=Enum: VentnMotPsonAvl  PortDimensions=1
  VentnActr08ResetSts_VentnActr08ResetSts : Inport  Port=268  OutDataTypeStr=Enum: VentnActr01ResetSts  PortDimensions=1
  VentnActr08SpclFct_VentnActr08SpclFct : Inport  Port=269  OutDataTypeStr=Enum: VentnActr01SpclFct  PortDimensions=1
  VentnActr08SpdSts_VentnActr08SpdSts : Inport  Port=270  OutDataTypeStr=Enum: VentnActr01SpdSts  PortDimensions=1
  VentnActr08TrqSts_VentnActr08TrqSts : Inport  Port=271  OutDataTypeStr=Enum: VentnMotUrgcUnlck  PortDimensions=1
  VentnActr08UrgcExist_VentnActr08UrgcExist : Inport  Port=272  OutDataTypeStr=Enum: VentnActr01UrgcExist  PortDimensions=1
  VentnActr08UrgcPson_VentnActr08UrgcPson : Inport  Port=273  OutDataTypeStr=Enum: VentnMotUrgcPson  PortDimensions=1
  VentnActr08UrgcSts_VentnActr08UrgcSts : Inport  Port=274  OutDataTypeStr=Enum: VentnMotUrgcUnlck  PortDimensions=1
  VentnActr08VoltErr_VentnActr08VoltErr : Inport  Port=275  OutDataTypeStr=Enum: VentnActr01VoltErr  PortDimensions=1
  VentnActr09ActPson_VentnActr09ActPson : Inport  Port=276  OutDataTypeStr=UInt16  PortDimensions=1
  VentnActr09ActPson_VentnActr09ActPson_IsUpdated : Inport  Port=277  OutDataTypeStr=boolean  PortDimensions=1
  VentnActr09BlckInd_VentnActr09BlckInd : Inport  Port=278  OutDataTypeStr=Enum: VentnActr01BlckInd  PortDimensions=1
  VentnActr09BlckSts_VentnActr09BlckSts : Inport  Port=279  OutDataTypeStr=Enum: VentnActr01BlckSts  PortDimensions=1
  VentnActr09BusAdr_VentnActr09BusAdr : Inport  Port=280  OutDataTypeStr=UInt8  PortDimensions=1
  VentnActr09CoilSts_VentnActr09CoilSts : Inport  Port=281  OutDataTypeStr=Enum: VentnMotUrgcUnlck  PortDimensions=1
  VentnActr09DrvDir_VentnActr09DrvDir : Inport  Port=282  OutDataTypeStr=Enum: VentnActr01DrvDir  PortDimensions=1
  VentnActr09ElecErr_VentnActr09ElecErr : Inport  Port=283  OutDataTypeStr=Enum: VentnActr01ElecErr  PortDimensions=1
  VentnActr09Mode_VentnActr09Mode : Inport  Port=284  OutDataTypeStr=Enum: VentnMotMode  PortDimensions=1
  VentnActr09MovDir_VentnActr09MovDir : Inport  Port=285  OutDataTypeStr=Enum: VentnMotDir  PortDimensions=1
  VentnActr09OvrTempErr_VentnActr09OvrTempErr : Inport  Port=286  OutDataTypeStr=Enum: VentnActr01OvrTempErr  PortDimensions=1
  VentnActr09PsonSts_VentnActr09PsonSts : Inport  Port=287  OutDataTypeStr=Enum: VentnMotPsonAvl  PortDimensions=1
  VentnActr09ResetSts_VentnActr09ResetSts : Inport  Port=288  OutDataTypeStr=Enum: VentnActr01ResetSts  PortDimensions=1
  VentnActr09SpclFct_VentnActr09SpclFct : Inport  Port=289  OutDataTypeStr=Enum: VentnActr01SpclFct  PortDimensions=1
  VentnActr09SpdSts_VentnActr09SpdSts : Inport  Port=290  OutDataTypeStr=Enum: VentnActr01SpdSts  PortDimensions=1
  VentnActr09TrqSts_VentnActr09TrqSts : Inport  Port=291  OutDataTypeStr=Enum: VentnMotUrgcUnlck  PortDimensions=1
  VentnActr09UrgcExist_VentnActr09UrgcExist : Inport  Port=292  OutDataTypeStr=Enum: VentnActr01UrgcExist  PortDimensions=1
  VentnActr09UrgcPson_VentnActr09UrgcPson : Inport  Port=293  OutDataTypeStr=Enum: VentnMotUrgcPson  PortDimensions=1
  VentnActr09UrgcSts_VentnActr09UrgcSts : Inport  Port=294  OutDataTypeStr=Enum: VentnMotUrgcUnlck  PortDimensions=1
  VentnActr09VoltErr_VentnActr09VoltErr : Inport  Port=295  OutDataTypeStr=Enum: VentnActr01VoltErr  PortDimensions=1
  VentnActr10ActPson_VentnActr10ActPson : Inport  Port=296  OutDataTypeStr=UInt16  PortDimensions=1
  VentnActr10ActPson_VentnActr10ActPson_IsUpdated : Inport  Port=297  OutDataTypeStr=boolean  PortDimensions=1
  VentnActr10BlckInd_VentnActr10BlckInd : Inport  Port=298  OutDataTypeStr=Enum: VentnActr01BlckInd  PortDimensions=1
  VentnActr10BlckSts_VentnActr10BlckSts : Inport  Port=299  OutDataTypeStr=Enum: VentnActr01BlckSts  PortDimensions=1
  VentnActr10BusAdr_VentnActr10BusAdr : Inport  Port=300  OutDataTypeStr=UInt8  PortDimensions=1
  VentnActr10CoilSts_VentnActr10CoilSts : Inport  Port=301  OutDataTypeStr=Enum: VentnMotUrgcUnlck  PortDimensions=1
  VentnActr10DrvDir_VentnActr10DrvDir : Inport  Port=302  OutDataTypeStr=Enum: VentnActr01DrvDir  PortDimensions=1
  VentnActr10ElecErr_VentnActr10ElecErr : Inport  Port=303  OutDataTypeStr=Enum: VentnActr01ElecErr  PortDimensions=1
  VentnActr10Mode_VentnActr10Mode : Inport  Port=304  OutDataTypeStr=Enum: VentnMotMode  PortDimensions=1
  VentnActr10MovDir_VentnActr10MovDir : Inport  Port=305  OutDataTypeStr=Enum: VentnMotDir  PortDimensions=1
  VentnActr10OvrTempErr_VentnActr10OvrTempErr : Inport  Port=306  OutDataTypeStr=Enum: VentnActr01OvrTempErr  PortDimensions=1
  VentnActr10PsonSts_VentnActr10PsonSts : Inport  Port=307  OutDataTypeStr=Enum: VentnMotPsonAvl  PortDimensions=1
  VentnActr10ResetSts_VentnActr10ResetSts : Inport  Port=308  OutDataTypeStr=Enum: VentnActr01ResetSts  PortDimensions=1
  VentnActr10SpclFct_VentnActr10SpclFct : Inport  Port=309  OutDataTypeStr=Enum: VentnActr01SpclFct  PortDimensions=1
  VentnActr10SpdSts_VentnActr10SpdSts : Inport  Port=310  OutDataTypeStr=Enum: VentnActr01SpdSts  PortDimensions=1
  VentnActr10TrqSts_VentnActr10TrqSts : Inport  Port=311  OutDataTypeStr=Enum: VentnMotUrgcUnlck  PortDimensions=1
  VentnActr10UrgcExist_VentnActr10UrgcExist : Inport  Port=312  OutDataTypeStr=Enum: VentnActr01UrgcExist  PortDimensions=1
  VentnActr10UrgcPson_VentnActr10UrgcPson : Inport  Port=313  OutDataTypeStr=Enum: VentnMotUrgcPson  PortDimensions=1
  VentnActr10UrgcSts_VentnActr10UrgcSts : Inport  Port=314  OutDataTypeStr=Enum: VentnMotUrgcUnlck  PortDimensions=1
  VentnActr10VoltErr_VentnActr10VoltErr : Inport  Port=315  OutDataTypeStr=Enum: VentnActr01VoltErr  PortDimensions=1
  VentnActr11ActPson_VentnActr11ActPson : Inport  Port=316  OutDataTypeStr=UInt16  PortDimensions=1
  VentnActr11ActPson_VentnActr11ActPson_IsUpdated : Inport  Port=317  OutDataTypeStr=boolean  PortDimensions=1
  VentnActr11BlckInd_VentnActr11BlckInd : Inport  Port=318  OutDataTypeStr=Enum: VentnActr01BlckInd  PortDimensions=1
  VentnActr11BlckSts_VentnActr11BlckSts : Inport  Port=319  OutDataTypeStr=Enum: VentnActr01BlckSts  PortDimensions=1
  VentnActr11BusAdr_VentnActr11BusAdr : Inport  Port=320  OutDataTypeStr=UInt8  PortDimensions=1
  VentnActr11CoilSts_VentnActr11CoilSts : Inport  Port=321  OutDataTypeStr=Enum: VentnMotUrgcUnlck  PortDimensions=1
  VentnActr11DrvDir_VentnActr11DrvDir : Inport  Port=322  OutDataTypeStr=Enum: VentnActr01DrvDir  PortDimensions=1
  VentnActr11ElecErr_VentnActr11ElecErr : Inport  Port=323  OutDataTypeStr=Enum: VentnActr01ElecErr  PortDimensions=1
  VentnActr11Mode_VentnActr11Mode : Inport  Port=324  OutDataTypeStr=Enum: VentnMotMode  PortDimensions=1
  VentnActr11MovDir_VentnActr11MovDir : Inport  Port=325  OutDataTypeStr=Enum: VentnMotDir  PortDimensions=1
  VentnActr11OvrTempErr_VentnActr11OvrTempErr : Inport  Port=326  OutDataTypeStr=Enum: VentnActr01OvrTempErr  PortDimensions=1
  VentnActr11PsonSts_VentnActr11PsonSts : Inport  Port=327  OutDataTypeStr=Enum: VentnMotPsonAvl  PortDimensions=1
  VentnActr11ResetSts_VentnActr11ResetSts : Inport  Port=328  OutDataTypeStr=Enum: VentnActr01ResetSts  PortDimensions=1
  VentnActr11SpclFct_VentnActr11SpclFct : Inport  Port=329  OutDataTypeStr=Enum: VentnActr01SpclFct  PortDimensions=1
  VentnActr11SpdSts_VentnActr11SpdSts : Inport  Port=330  OutDataTypeStr=Enum: VentnActr01SpdSts  PortDimensions=1
  VentnActr11TrqSts_VentnActr11TrqSts : Inport  Port=331  OutDataTypeStr=Enum: VentnMotUrgcUnlck  PortDimensions=1
  VentnActr11UrgcExist_VentnActr11UrgcExist : Inport  Port=332  OutDataTypeStr=Enum: VentnActr01UrgcExist  PortDimensions=1
  VentnActr11UrgcPson_VentnActr11UrgcPson : Inport  Port=333  OutDataTypeStr=Enum: VentnMotUrgcPson  PortDimensions=1
  VentnActr11UrgcSts_VentnActr11UrgcSts : Inport  Port=334  OutDataTypeStr=Enum: VentnMotUrgcUnlck  PortDimensions=1
  VentnActr11VoltErr_VentnActr11VoltErr : Inport  Port=335  OutDataTypeStr=Enum: VentnActr01VoltErr  PortDimensions=1
  VentnActr12ActPson_VentnActr12ActPson : Inport  Port=336  OutDataTypeStr=UInt16  PortDimensions=1
  VentnActr12ActPson_VentnActr12ActPson_IsUpdated : Inport  Port=337  OutDataTypeStr=boolean  PortDimensions=1
  VentnActr12BlckInd_VentnActr12BlckInd : Inport  Port=338  OutDataTypeStr=Enum: VentnActr01BlckInd  PortDimensions=1
  VentnActr12BlckSts_VentnActr12BlckSts : Inport  Port=339  OutDataTypeStr=Enum: VentnActr01BlckSts  PortDimensions=1
  VentnActr12BusAdr_VentnActr12BusAdr : Inport  Port=340  OutDataTypeStr=UInt8  PortDimensions=1
  VentnActr12CoilSts_VentnActr12CoilSts : Inport  Port=341  OutDataTypeStr=Enum: VentnMotUrgcUnlck  PortDimensions=1
  VentnActr12DrvDir_VentnActr12DrvDir : Inport  Port=342  OutDataTypeStr=Enum: VentnActr01DrvDir  PortDimensions=1
  VentnActr12ElecErr_VentnActr12ElecErr : Inport  Port=343  OutDataTypeStr=Enum: VentnActr01ElecErr  PortDimensions=1
  VentnActr12Mode_VentnActr12Mode : Inport  Port=344  OutDataTypeStr=Enum: VentnMotMode  PortDimensions=1
  VentnActr12MovDir_VentnActr12MovDir : Inport  Port=345  OutDataTypeStr=Enum: VentnMotDir  PortDimensions=1
  VentnActr12OvrTempErr_VentnActr12OvrTempErr : Inport  Port=346  OutDataTypeStr=Enum: VentnActr01OvrTempErr  PortDimensions=1
  VentnActr12PsonSts_VentnActr12PsonSts : Inport  Port=347  OutDataTypeStr=Enum: VentnMotPsonAvl  PortDimensions=1
  VentnActr12ResetSts_VentnActr12ResetSts : Inport  Port=348  OutDataTypeStr=Enum: VentnActr01ResetSts  PortDimensions=1
  VentnActr12SpclFct_VentnActr12SpclFct : Inport  Port=349  OutDataTypeStr=Enum: VentnActr01SpclFct  PortDimensions=1
  VentnActr12SpdSts_VentnActr12SpdSts : Inport  Port=350  OutDataTypeStr=Enum: VentnActr01SpdSts  PortDimensions=1